simulink model "SyDdemo"  (Simulink 7.0)
settings: Solver=FixedStepDiscrete  StartTime=0.0  StopTime=10.0  FixedStep=0.01  RelTol=1e-3
model workspace (MAT data in the model file):
  air_esti_error : Simulink.Signal (value not decoded)
  atmo_pressure : Simulink.Signal (value not decoded)
  battery_volt : Simulink.Signal (value not decoded)
  control_pwm : Simulink.Signal (value not decoded)
  control_volt : Simulink.Signal (value not decoded)
  controller_error : Simulink.Signal (value not decoded)
  controller_error_lim : Simulink.Signal (value not decoded)
  controller_r0_out : Simulink.Signal (value not decoded)
  controller_r1_out : Simulink.Signal (value not decoded)
  controller_r2_out : Simulink.Signal (value not decoded)
  controller_r3_out : Simulink.Signal (value not decoded)
  controller_r_out : Simulink.Signal (value not decoded)
  controller_s0_out : Simulink.Signal (value not decoded)
  controller_s1_out : Simulink.Signal (value not decoded)
  controller_s2_out : Simulink.Signal (value not decoded)
  controller_s_out : Simulink.Signal (value not decoded)
  controller_s_out_prev : Simulink.Signal (value not decoded)
  controller_t0_out : Simulink.Signal (value not decoded)
  controller_t1_out : Simulink.Signal (value not decoded)
  controller_t2_out : Simulink.Signal (value not decoded)
  controller_t_out : Simulink.Signal (value not decoded)
  coolant_temp : Simulink.Signal (value not decoded)
  diag_control_ena : Simulink.Signal (value not decoded)
  diag_sp_ena : Simulink.Signal (value not decoded)
  engine_speed : Simulink.Signal (value not decoded)
  engine_status : Simulink.Signal (value not decoded)
  friction_comp_volt : Simulink.Signal (value not decoded)
  global_control_error : Simulink.Signal (value not decoded)
  pos_dmnd_raw : Simulink.Signal (value not decoded)
  pos_feedback : Simulink.Signal (value not decoded)
  pos_offset : Simulink.Signal (value not decoded)
  pos_relative : Simulink.Signal (value not decoded)
  pos_setpoint : Simulink.Signal (value not decoded)
  COR_COOLANT_TEMP_XDAT : Simulink.Parameter (value not decoded)
  COR_COOLANT_TEMP_YDAT : Simulink.Parameter (value not decoded)
  COR_ATMO_PRESSURE_XDAT : Simulink.Parameter (value not decoded)
  COR_ATMO_PRESSURE_YDAT : Simulink.Parameter (value not decoded)
  COR_AIR_ESTI_ERROR_GAIN : Simulink.Parameter (value not decoded)
  CONTROLLER_T2 : Simulink.Parameter (value not decoded)
  CONTROLLER_T1 : Simulink.Parameter (value not decoded)
  CONTROLLER_T0 : Simulink.Parameter (value not decoded)
  CONTROLLER_SETPONT_SIGN : Simulink.Parameter (value not decoded)
  CONTROLLER_R3 : Simulink.Parameter (value not decoded)
  CONTROLLER_R2 : Simulink.Parameter (value not decoded)
  CONTROLLER_R1 : Simulink.Parameter (value not decoded)
  CONTROLLER_SIGN : Simulink.Parameter (value not decoded)
  CONTROLLER_R0 : Simulink.Parameter (value not decoded)
  CONTROLLER_S2 : Simulink.Parameter (value not decoded)
  CONTROLLER_S1 : Simulink.Parameter (value not decoded)
  CONTROLLER_S0 : Simulink.Parameter (value not decoded)
  CONTROLLER_ERROR_LIM : Simulink.Parameter (value not decoded)
  FRICTION_DYN_COMP_VOLT : Simulink.Parameter (value not decoded)
  FRICTION_COMP_THR : Simulink.Parameter (value not decoded)
  FRICTION_STATIC_COMP_VOLT : Simulink.Parameter (value not decoded)
  RUNNING : Simulink.Parameter (value not decoded)
PreLoadFcn:
  | addpath(genpath(fileparts(get_param(gcs,'FileName'))));
libraries: simulink x15, SyDdemoChildren x2
settings Model: Creator=Aurelien PROST  LastModifiedBy=LeanPulse
settings Model: ModifiedHistory=|
  | <syd:extradata xmlns:syd="http://www.leanpulse.com/schemas/syd/2011/core" owner="LeanPulse" ref="SPEC0001" title="SyD Demo Model"><syd:version author="Aurélien PROST" date="10 Jan 2011" num="1.0" opt1="a.prost@leanpulse.com" opt2="">This specification has been created for demo purpose in order to highlight the main features of the LeanPulse System Document Generator (SyD).
  | 
  | (c) LeanPulse 2011</syd:version><syd:version author="Aurélien PROST" date="11 Jan 2011" num="2.0" opt1="a.prost@leanpulse.com" opt2="">You can edit this history by using the document metadata editor available in the file menu of your model.</syd:version></syd:extradata>
settings OptimizationCC: InitFltsAndDblsToZero=off
settings DebuggingCC: StrictBusMsg=ErrorLevel1

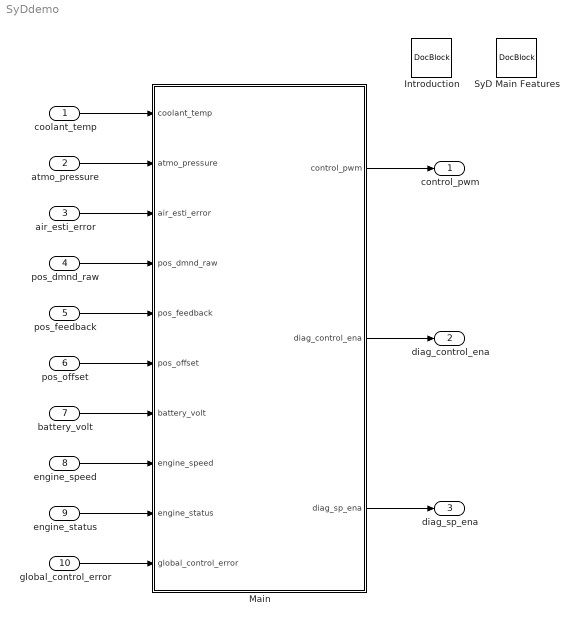
== system SyDdemo ==
  coolant_temp : Inport
  atmo_pressure : Inport  Port=2
  air_esti_error : Inport  Port=3
  pos_dmnd_raw : Inport  Port=4
  pos_feedback : Inport  Port=5
  pos_offset : Inport  Port=6
  battery_volt : Inport  Port=7
  engine_speed : Inport  Port=8
  engine_status : Inport  Port=9
  global_control_error : Inport  Port=10
  Introduction : simulink/Model-Wide Utilities/DocBlock  SystemSampleTime=-1  DocumentType=RTF
  Main : Subsystem
  SyD Main Features : simulink/Model-Wide Utilities/DocBlock  SystemSampleTime=-1  DocumentType=RTF
  control_pwm : Outport
  diag_control_ena : Outport  Port=2
  diag_sp_ena : Outport  Port=3
  wires:
  coolant_temp/1 -> Main/1(coolant_temp)
  atmo_pressure/1 -> Main/2(atmo_pressure)
  air_esti_error/1 -> Main/3(air_esti_error)
  pos_dmnd_raw/1 -> Main/4(pos_dmnd_raw)
  pos_feedback/1 -> Main/5(pos_feedback)
  pos_offset/1 -> Main/6(pos_offset)
  battery_volt/1 -> Main/7(battery_volt)
  engine_speed/1 -> Main/8(engine_speed)
  engine_status/1 -> Main/9(engine_status)
  global_control_error/1 -> Main/10(global_control_error)
  Main/1(control_pwm) -> control_pwm/1
  Main/2(diag_control_ena) -> diag_control_ena/1
  Main/3(diag_sp_ena) -> diag_sp_ena/1

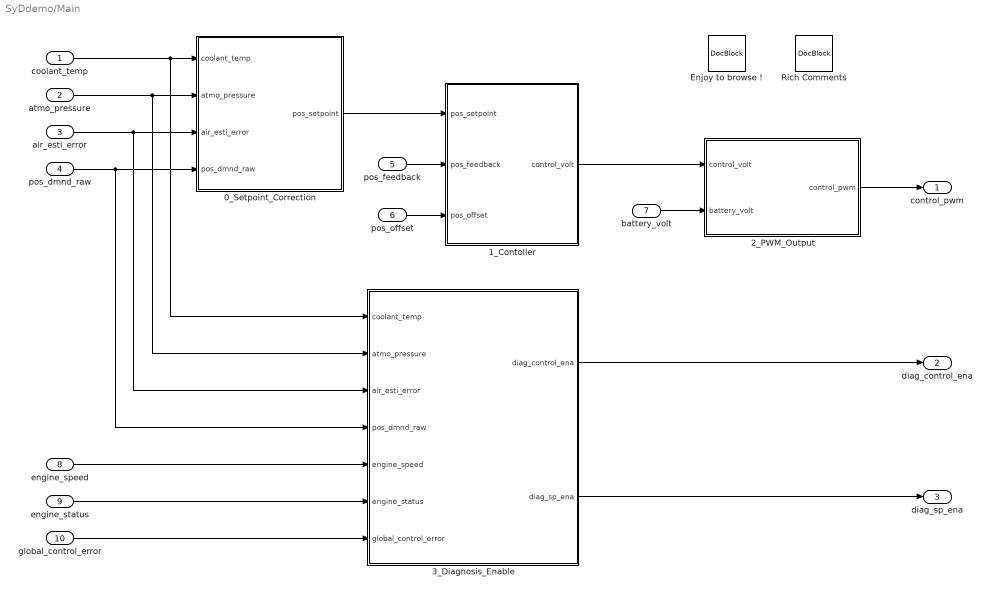
== system SyDdemo/Main ==
  coolant_temp : Inport
  atmo_pressure : Inport  Port=2
  air_esti_error : Inport  Port=3
  pos_dmnd_raw : Inport  Port=4
  pos_feedback : Inport  Port=5
  pos_offset : Inport  Port=6
  battery_volt : Inport  Port=7
  engine_speed : Inport  Port=8
  engine_status : Inport  Port=9
  global_control_error : Inport  Port=10
  0_Setpoint_Correction : Subsystem  Description="This comment has been written in the description field of the SubSystem \"0_Setpoint_Correction\"."
  1_Contoller : Subsystem
  2_PWM_Output : Subsystem
  3_Diagnosis_Enable : Subsystem
  Enjoy to browse ! : simulink/Model-Wide Utilities/DocBlock  SystemSampleTime=-1  DocumentType=RTF
  Rich Comments : simulink/Model-Wide Utilities/DocBlock  SystemSampleTime=-1  DocumentType=RTF
  control_pwm : Outport
  diag_control_ena : Outport  Port=2
  diag_sp_ena : Outport  Port=3
  wires:
  0_Setpoint_Correction/1(pos_setpoint) -> 1_Contoller/1(pos_setpoint)
  coolant_temp/1 -> 0_Setpoint_Correction/1(coolant_temp), 3_Diagnosis_Enable/1(coolant_temp)
  atmo_pressure/1 -> 0_Setpoint_Correction/2(atmo_pressure), 3_Diagnosis_Enable/2(atmo_pressure)
  air_esti_error/1 -> 0_Setpoint_Correction/3(air_esti_error), 3_Diagnosis_Enable/3(air_esti_error)
  pos_dmnd_raw/1 -> 0_Setpoint_Correction/4(pos_dmnd_raw), 3_Diagnosis_Enable/4(pos_dmnd_raw)
  pos_feedback/1 -> 1_Contoller/2(pos_feedback)
  battery_volt/1 -> 2_PWM_Output/2(battery_volt)
  engine_status/1 -> 3_Diagnosis_Enable/6(engine_status)
  global_control_error/1 -> 3_Diagnosis_Enable/7(global_control_error)
  engine_speed/1 -> 3_Diagnosis_Enable/5(engine_speed)
  2_PWM_Output/1(control_pwm) -> control_pwm/1
  3_Diagnosis_Enable/1(diag_control_ena) -> diag_control_ena/1
  3_Diagnosis_Enable/2(diag_sp_ena) -> diag_sp_ena/1
  1_Contoller/1(control_volt) -> 2_PWM_Output/1(control_volt)
  pos_offset/1 -> 1_Contoller/3(pos_offset)

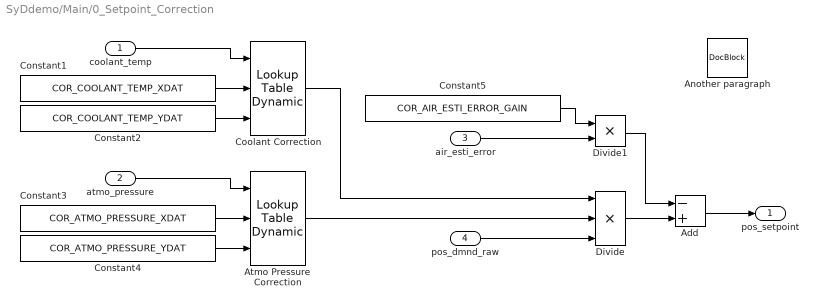
== system SyDdemo/Main/0_Setpoint_Correction ==
  coolant_temp : Inport
  atmo_pressure : Inport  Port=2
  air_esti_error : Inport  Port=3
  pos_dmnd_raw : Inport  Port=4
  Add : Sum  Inputs=-+
  Another paragraph : simulink/Model-Wide Utilities/DocBlock  SystemSampleTime=-1  DocumentType=RTF
  Atmo Pressure Correction : simulink/Lookup Tables/Lookup Table Dynamic  LookUpMeth=Interpolation-Use End Values  OutputDataTypeScalingMode=Specify via dialog  OutDataType=fixdt('double')  OutScaling=2^-10  DoSatur=off
  Constant1 : Constant  Value=COR_COOLANT_TEMP_XDAT
  Constant2 : Constant  Value=COR_COOLANT_TEMP_YDAT
  Constant3 : Constant  Value=COR_ATMO_PRESSURE_XDAT
  Constant4 : Constant  Value=COR_ATMO_PRESSURE_YDAT
  Constant5 : Constant  Value=COR_AIR_ESTI_ERROR_GAIN
  Coolant Correction : simulink/Lookup Tables/Lookup Table Dynamic  LookUpMeth=Interpolation-Use End Values  OutputDataTypeScalingMode=Specify via dialog  OutDataType=fixdt('double')  OutScaling=2^-10  DoSatur=off
  Divide : Product  Inputs=***
  Divide1 : Product  Inputs=**
  pos_setpoint : Outport
  wires:
  coolant_temp/1 -> Coolant Correction/1
  Constant1/1 -> Coolant Correction/2
  Constant2/1 -> Coolant Correction/3
  atmo_pressure/1 -> Atmo Pressure Correction/1
  Constant3/1 -> Atmo Pressure Correction/2
  Constant4/1 -> Atmo Pressure Correction/3
  Coolant Correction/1 -> Divide/1
  Atmo Pressure Correction/1 -> Divide/2
  pos_dmnd_raw/1 -> Divide/3
  air_esti_error/1 -> Divide1/2
  Constant5/1 -> Divide1/1
  Divide/1 -> Add/2
  Divide1/1 -> Add/1
  Add/1 -> pos_setpoint/1

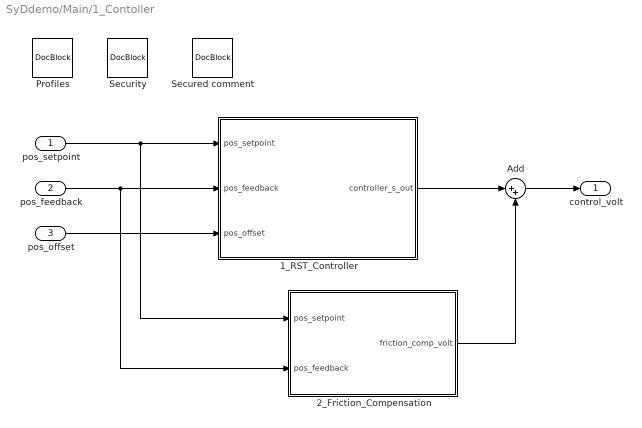
== system SyDdemo/Main/1_Contoller ==
  pos_setpoint : Inport
  pos_feedback : Inport  Port=2
  pos_offset : Inport  Port=3
  1_RST_Controller : Subsystem
  2_Friction_Compensation : Subsystem
  Add : Sum  Inputs=|++
  Profiles : simulink/Model-Wide Utilities/DocBlock  SystemSampleTime=-1  DocumentType=RTF
  Secured comment : simulink/Model-Wide Utilities/DocBlock  Description="<syd:extradata xmlns:syd=\"http://www.leanpulse.com/schemas/syd/2011/core\" security=\"2\"/>"  SystemSampleTime=-1  DocumentType=RTF
  Security : simulink/Model-Wide Utilities/DocBlock  SystemSampleTime=-1  DocumentType=RTF
  control_volt : Outport
  wires:
  2_Friction_Compensation/1(friction_comp_volt) -> Add/2
  1_RST_Controller/1(controller_s_out) -> Add/1
  Add/1 -> control_volt/1
  pos_feedback/1 -> 1_RST_Controller/2(pos_feedback), 2_Friction_Compensation/2(pos_feedback)
  pos_setpoint/1 -> 1_RST_Controller/1(pos_setpoint), 2_Friction_Compensation/1(pos_setpoint)
  pos_offset/1 -> 1_RST_Controller/3(pos_offset)

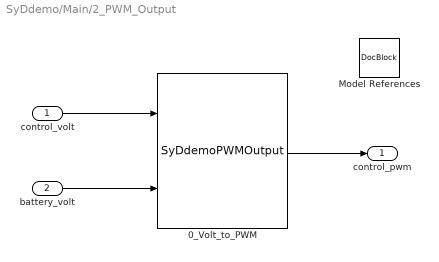
== system SyDdemo/Main/2_PWM_Output ==
  control_volt : Inport
  battery_volt : Inport  Port=2
  0_Volt_to_PWM : SyDdemoChildren/SyDdemoPWMOutput  Tag=SyDlink  SystemSampleTime=-1
  Model References : simulink/Model-Wide Utilities/DocBlock  SystemSampleTime=-1  DocumentType=RTF
  control_pwm : Outport
  wires:
  control_volt/1 -> 0_Volt_to_PWM/1
  battery_volt/1 -> 0_Volt_to_PWM/2
  0_Volt_to_PWM/1 -> control_pwm/1

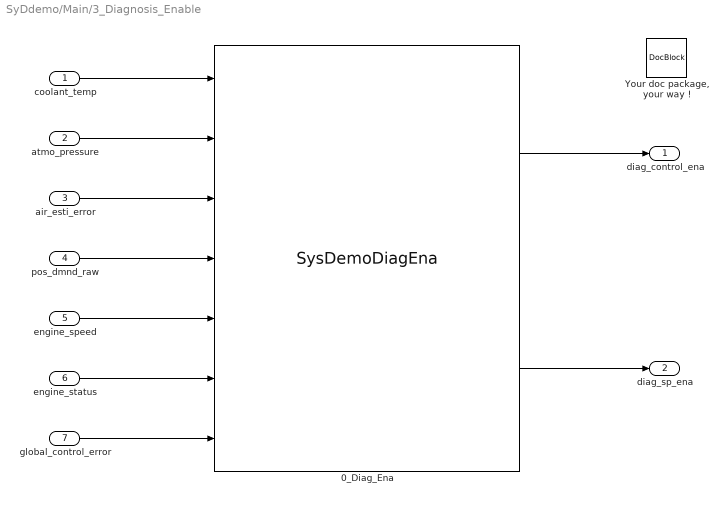
== system SyDdemo/Main/3_Diagnosis_Enable ==
  coolant_temp : Inport
  atmo_pressure : Inport  Port=2
  air_esti_error : Inport  Port=3
  pos_dmnd_raw : Inport  Port=4
  engine_speed : Inport  Port=5
  engine_status : Inport  Port=6
  global_control_error : Inport  Port=7
  0_Diag_Ena : SyDdemoChildren/SysDemoDiagEna  Tag=SyDlink  SystemSampleTime=-1
  "Your doc package, your way !" : simulink/Model-Wide Utilities/DocBlock  SystemSampleTime=-1  DocumentType=RTF
  diag_control_ena : Outport
  diag_sp_ena : Outport  Port=2
  wires:
  0_Diag_Ena/1 -> diag_control_ena/1
  0_Diag_Ena/2 -> diag_sp_ena/1
  coolant_temp/1 -> 0_Diag_Ena/1
  atmo_pressure/1 -> 0_Diag_Ena/2
  air_esti_error/1 -> 0_Diag_Ena/3
  pos_dmnd_raw/1 -> 0_Diag_Ena/4
  engine_speed/1 -> 0_Diag_Ena/5
  engine_status/1 -> 0_Diag_Ena/6
  global_control_error/1 -> 0_Diag_Ena/7

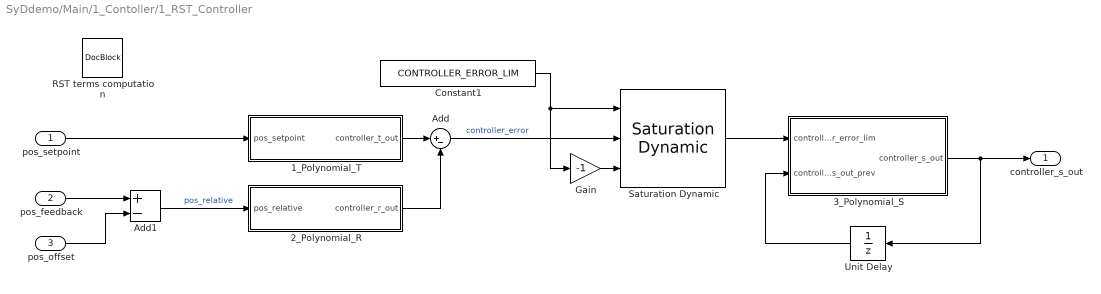
== system SyDdemo/Main/1_Contoller/1_RST_Controller ==
  pos_setpoint : Inport
  pos_feedback : Inport  Port=2
  pos_offset : Inport  Port=3
  1_Polynomial_T : Subsystem
  2_Polynomial_R : Subsystem
  3_Polynomial_S : Subsystem
  Add : Sum  Inputs=|+-
  Add1 : Sum  Inputs=+-
  Constant1 : Constant  Value=CONTROLLER_ERROR_LIM
  Gain : Gain  Gain=-1
  RST terms computation : simulink/Model-Wide Utilities/DocBlock  SystemSampleTime=-1  DocumentType=RTF
  Saturation Dynamic : simulink/Discontinuities/Saturation Dynamic  SystemSampleTime=-1  OutMin=[]  OutMax=[]  OutDataType=fixdt(1, 16)  OutScaling=2^-10  DoSatur=off
  Unit Delay : UnitDelay  Orientation=left  SampleTime=-1
      settings: AttributesFormatString=Init=%<X0>
  controller_s_out : Outport
  wires:
  pos_feedback/1 -> Add1/1
  pos_offset/1 -> Add1/2
  Add1/1 -> 2_Polynomial_R/1(pos_relative)  "pos_relative"
  pos_setpoint/1 -> 1_Polynomial_T/1(pos_setpoint)
  1_Polynomial_T/1(controller_t_out) -> Add/1
  2_Polynomial_R/1(controller_r_out) -> Add/2
  Add/1 -> Saturation Dynamic/2  "controller_error"
  Constant1/1 -> Saturation Dynamic/1, Gain/1
  Gain/1 -> Saturation Dynamic/3
  Saturation Dynamic/1 -> 3_Polynomial_S/1(controller_error_lim)
  Unit Delay/1 -> 3_Polynomial_S/2(controller_s_out_prev)
  3_Polynomial_S/1(controller_s_out) -> controller_s_out/1, Unit Delay/1

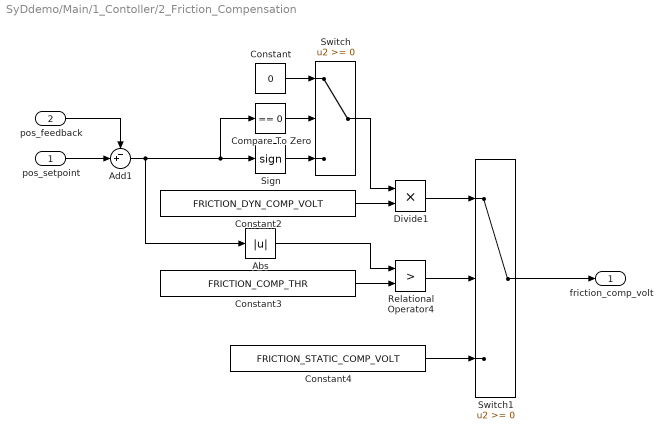
== system SyDdemo/Main/1_Contoller/2_Friction_Compensation ==
  pos_setpoint : Inport
  pos_feedback : Inport  Port=2
  Abs : Abs
  Add1 : Sum  Inputs=-+|
  Compare To Zero : simulink/Logic and Bit Operations/Compare To Zero  SystemSampleTime=-1  relop===  LogicOutDataTypeMode=boolean
  Constant : Constant  Value=0
  Constant2 : Constant  Value=FRICTION_DYN_COMP_VOLT
  Constant3 : Constant  Value=FRICTION_COMP_THR
  Constant4 : Constant  Value=FRICTION_STATIC_COMP_VOLT
  Divide1 : Product  Inputs=**
  Relational Operator4 : RelationalOperator  Operator=>  LogicOutDataTypeMode=boolean
  Sign : Signum
  Switch : Switch
  Switch1 : Switch
  friction_comp_volt : Outport
  wires:
  Add1/1 -> Sign/1, Compare To Zero/1, Abs/1
  Compare To Zero/1 -> Switch/2
  Sign/1 -> Switch/3
  Constant/1 -> Switch/1
  Constant2/1 -> Divide1/2
  Switch/1 -> Divide1/1
  pos_setpoint/1 -> Add1/2
  pos_feedback/1 -> Add1/1
  Constant3/1 -> Relational Operator4/2
  Abs/1 -> Relational Operator4/1
  Relational Operator4/1 -> Switch1/2
  Divide1/1 -> Switch1/1
  Constant4/1 -> Switch1/3
  Switch1/1 -> friction_comp_volt/1

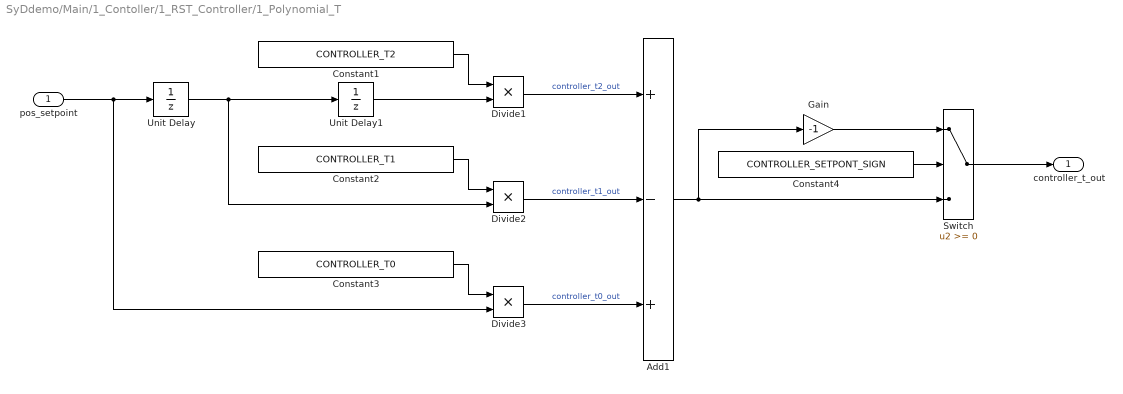
== system SyDdemo/Main/1_Contoller/1_RST_Controller/1_Polynomial_T ==
  pos_setpoint : Inport
  Add1 : Sum  Inputs=+-+
  Constant1 : Constant  Value=CONTROLLER_T2
  Constant2 : Constant  Value=CONTROLLER_T1
  Constant3 : Constant  Value=CONTROLLER_T0
  Constant4 : Constant  Value=CONTROLLER_SETPONT_SIGN
  Divide1 : Product  Inputs=**
  Divide2 : Product  Inputs=**
  Divide3 : Product  Inputs=**
  Gain : Gain  Gain=-1
  Switch : Switch
  Unit Delay : UnitDelay  SampleTime=-1
      settings: AttributesFormatString=Init=%<X0>
  Unit Delay1 : UnitDelay  SampleTime=-1
      settings: AttributesFormatString=Init=%<X0>
  controller_t_out : Outport
  wires:
  Constant1/1 -> Divide1/1
  Unit Delay1/1 -> Divide1/2
  Constant2/1 -> Divide2/1
  Constant3/1 -> Divide3/1
  Unit Delay/1 -> Unit Delay1/1, Divide2/2
  pos_setpoint/1 -> Unit Delay/1, Divide3/2
  Divide2/1 -> Add1/2  "controller_t1_out"
  Divide1/1 -> Add1/1  "controller_t2_out"
  Divide3/1 -> Add1/3  "controller_t0_out"
  Switch/1 -> controller_t_out/1
  Add1/1 -> Switch/3, Gain/1
  Constant4/1 -> Switch/2
  Gain/1 -> Switch/1

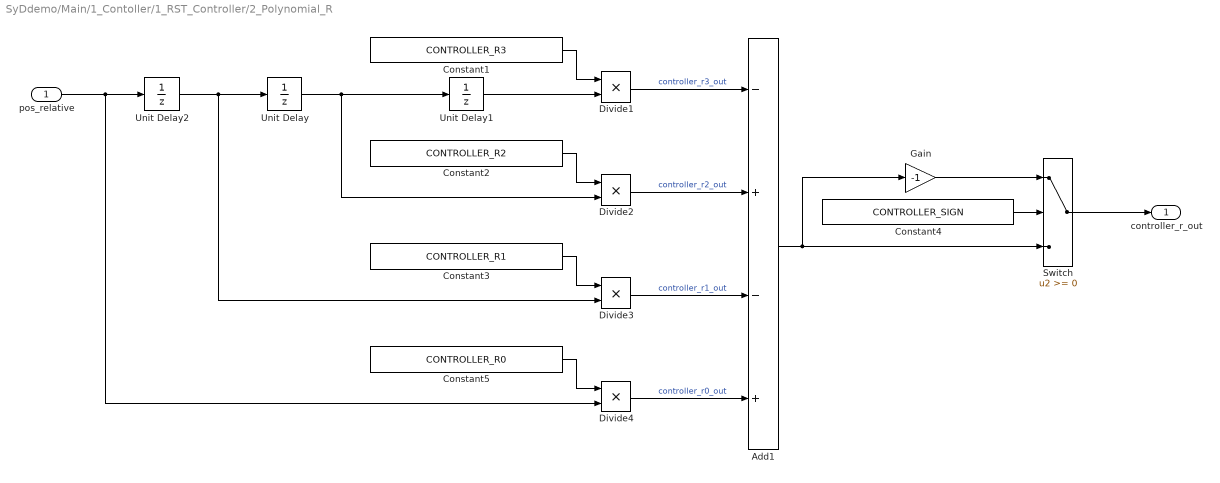
== system SyDdemo/Main/1_Contoller/1_RST_Controller/2_Polynomial_R ==
  pos_relative : Inport
  Add1 : Sum  Inputs=-+-+
  Constant1 : Constant  Value=CONTROLLER_R3
  Constant2 : Constant  Value=CONTROLLER_R2
  Constant3 : Constant  Value=CONTROLLER_R1
  Constant4 : Constant  Value=CONTROLLER_SIGN
  Constant5 : Constant  Value=CONTROLLER_R0
  Divide{1..4} (4 alike) : Product  Inputs=**
  Gain : Gain  Gain=-1
  Switch : Switch
  Unit Delay : UnitDelay  SampleTime=-1
      settings: AttributesFormatString=Init=%<X0>
  Unit Delay1 : UnitDelay  SampleTime=-1
      settings: AttributesFormatString=Init=%<X0>
  Unit Delay2 : UnitDelay  SampleTime=-1
      settings: AttributesFormatString=Init=%<X0>
  controller_r_out : Outport
  wires:
  Gain/1 -> Switch/1
  Constant4/1 -> Switch/2
  Add1/1 -> Gain/1, Switch/3
  Switch/1 -> controller_r_out/1
  Divide3/1 -> Add1/3  "controller_r1_out"
  Divide1/1 -> Add1/1  "controller_r3_out"
  Divide2/1 -> Add1/2  "controller_r2_out"
  pos_relative/1 -> Unit Delay2/1, Divide4/2
  Unit Delay/1 -> Divide2/2, Unit Delay1/1
  Constant3/1 -> Divide3/1
  Constant2/1 -> Divide2/1
  Unit Delay1/1 -> Divide1/2
  Constant1/1 -> Divide1/1
  Divide4/1 -> Add1/4  "controller_r0_out"
  Constant5/1 -> Divide4/1
  Unit Delay2/1 -> Divide3/2, Unit Delay/1

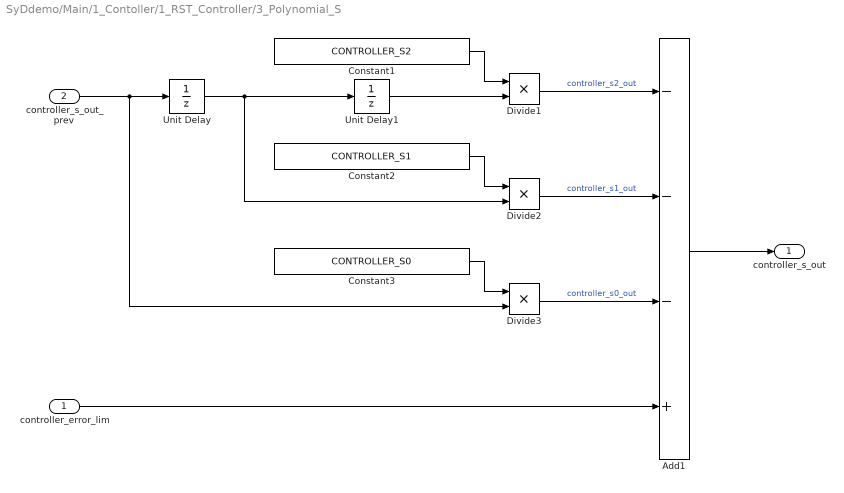
== system SyDdemo/Main/1_Contoller/1_RST_Controller/3_Polynomial_S ==
  controller_error_lim : Inport
  controller_s_out_prev : Inport  Port=2
  Add1 : Sum  Inputs=---+
  Constant1 : Constant  Value=CONTROLLER_S2
  Constant2 : Constant  Value=CONTROLLER_S1
  Constant3 : Constant  Value=CONTROLLER_S0
  Divide1 : Product  Inputs=**
  Divide2 : Product  Inputs=**
  Divide3 : Product  Inputs=**
  Unit Delay : UnitDelay  SampleTime=-1
      settings: AttributesFormatString=Init=%<X0>
  Unit Delay1 : UnitDelay  SampleTime=-1
      settings: AttributesFormatString=Init=%<X0>
  controller_s_out : Outport
  wires:
  Constant1/1 -> Divide1/1
  Unit Delay1/1 -> Divide1/2
  Constant2/1 -> Divide2/1
  Constant3/1 -> Divide3/1
  Unit Delay/1 -> Unit Delay1/1, Divide2/2
  controller_s_out_prev/1 -> Unit Delay/1, Divide3/2
  Divide2/1 -> Add1/2  "controller_s1_out"
  Divide1/1 -> Add1/1  "controller_s2_out"
  Divide3/1 -> Add1/3  "controller_s0_out"
  controller_error_lim/1 -> Add1/4
  Add1/1 -> controller_s_out/1
